simulink library "inp"  (Simulink 8.2)
settings: Solver=ode45  SolverMode=SingleTasking  StartTime=0.0  StopTime=10.0  RelTol=1e-3
undefined symbols (not defined in the file or in its folder): C_TICK_TIME_S, ENUM_FAULTSTATE_FAIL, ENUM_FAULTSTATE_INDETERMINATE, ENUM_FAULTSTATE_PASS, ENUM_KEYSWITCH_ON, FALSE, KINP_ABS1CANCommuOk_flg_ovrdflg, KINP_ABS1CANCommuOk_flg_ovrdval, KINP_ABS1CANPeriodTime_s, KINP_ABS1CANRolCntOk_flg_ovrdflg, KINP_ABS1CANRolCntOk_flg_ovrdval, KINP_ABS2CANChkSumOk_flg_ovrdflg, KINP_ABS2CANChkSumOk_flg_ovrdval, KINP_ABS2CANCommuOk_flg_ovrdflg, KINP_ABS2CANCommuOk_flg_ovrdval, KINP_ABS2CANPeriodTime_s, KINP_ABS2CANRolCntOk_flg_ovrdflg, KINP_ABS2CANRolCntOk_flg_ovrdval, KINP_ABS3CANCommuOk_flg_ovrdflg, KINP_ABS3CANCommuOk_flg_ovrdval, KINP_ABS4CANCommuOk_flg_ovrdflg, KINP_ABS4CANCommuOk_flg_ovrdval, KINP_ABSActive_flg_defval, KINP_ABSActive_flg_invalid, KINP_ABSActive_flg_ovrdflg, KINP_ABSActive_flg_ovrdval, KINP_ABSActive_flg_setvalid, KINP_ABSActive_flg_srvcflg, KINP_ABSActive_flg_srvcval, KINP_ABSCANAlive_flg_ovrdflg, KINP_ABSCANAlive_flg_ovrdval, KINP_ABSCANCommuOk_flg_ovrdflg, KINP_ABSCANCommuOk_flg_ovrdval, KINP_ABSCANValidOk_flg_ovrdflg, KINP_ABSCANValidOk_flg_ovrdval, KINP_ABSFault_flg_defval, KINP_ABSFault_flg_invalid, KINP_ABSFault_flg_ovrdflg, KINP_ABSFault_flg_ovrdval, KINP_ABSFault_flg_setvalid, KINP_ABSFault_flg_srvcflg, KINP_ABSFault_flg_srvcval, KINP_AC1CANCommuOk_flg_ovrdflg, KINP_AC1CANCommuOk_flg_ovrdval, KINP_ACCANAlive_flg_ovrdflg, KINP_ACCANAlive_flg_ovrdval, KINP_ACCANCommuOk_flg_ovrdflg, KINP_ACCANCommuOk_flg_ovrdval, KINP_ACCANValidOk_flg_ovrdflg, KINP_ACCANValidOk_flg_ovrdval, KINP_ACCCancelH_flg_defval, KINP_ACCCancelH_flg_invalid, KINP_ACCCancelH_flg_ovrdflg, KINP_ACCCancelH_flg_ovrdval, KINP_ACCCancelH_flg_setvalid, KINP_ACCCancelH_flg_srvcflg, KINP_ACCCancelH_flg_srvcval, KINP_AccelPedalPosn_pct_defval, KINP_AccelPedalPosn_pct_gain, KINP_AccelPedalPosn_pct_invalid ... (+1866)
libraries: inp x192, hcu_lib x34, simulink x33
(part 13 of 21)

== system inp/INP/HMI_signals/LFCPilotOnH ==
  signal_in : Inport
  msg_valid : Inport  Port=2
  Active_fault_flg : Inport  Port=3
  can_signal_process_wio_validity : inp/can_signal_process_wio_validity
  defval : Constant  Value=KINP_LFCPilotOnH_flg_defval
  invalid : Constant  Value=KINP_LFCPilotOnH_flg_invalid
  ovrdflg : Constant  Value=KINP_LFCPilotOnH_flg_ovrdflg
  ovrdval : Constant  Value=KINP_LFCPilotOnH_flg_ovrdval
  setvalid : Constant  Value=KINP_LFCPilotOnH_flg_setvalid
  srvcflg : Constant  Value=KINP_LFCPilotOnH_flg_srvcflg
  srvcval : Constant  Value=KINP_LFCPilotOnH_flg_srvcval
  signal_out : Outport
  signal_valid_out : Outport  Port=2
  wires:
  can_signal_process_wio_validity/1 -> signal_out/1
  can_signal_process_wio_validity/2 -> signal_valid_out/1
  signal_in/1 -> can_signal_process_wio_validity/1
  msg_valid/1 -> can_signal_process_wio_validity/2
  defval/1 -> can_signal_process_wio_validity/4
  invalid/1 -> can_signal_process_wio_validity/5
  setvalid/1 -> can_signal_process_wio_validity/6
  ovrdflg/1 -> can_signal_process_wio_validity/7
  ovrdval/1 -> can_signal_process_wio_validity/8
  srvcflg/1 -> can_signal_process_wio_validity/9
  srvcval/1 -> can_signal_process_wio_validity/10
  Active_fault_flg/1 -> can_signal_process_wio_validity/3

== system inp/INP/HMI_signals/LKAEnableH ==
  signal_in : Inport
  msg_valid : Inport  Port=2
  Active_fault_flg : Inport  Port=3
  can_signal_process_wio_validity : inp/can_signal_process_wio_validity
  defval : Constant  Value=KINP_LKAEnableH_flg_defval
  invalid : Constant  Value=KINP_LKAEnableH_flg_invalid
  ovrdflg : Constant  Value=KINP_LKAEnableH_flg_ovrdflg
  ovrdval : Constant  Value=KINP_LKAEnableH_flg_ovrdval
  setvalid : Constant  Value=KINP_LKAEnableH_flg_setvalid
  srvcflg : Constant  Value=KINP_LKAEnableH_flg_srvcflg
  srvcval : Constant  Value=KINP_LKAEnableH_flg_srvcval
  signal_out : Outport
  signal_valid_out : Outport  Port=2
  wires:
  Active_fault_flg/1 -> can_signal_process_wio_validity/3
  srvcval/1 -> can_signal_process_wio_validity/10
  srvcflg/1 -> can_signal_process_wio_validity/9
  ovrdval/1 -> can_signal_process_wio_validity/8
  ovrdflg/1 -> can_signal_process_wio_validity/7
  setvalid/1 -> can_signal_process_wio_validity/6
  invalid/1 -> can_signal_process_wio_validity/5
  defval/1 -> can_signal_process_wio_validity/4
  msg_valid/1 -> can_signal_process_wio_validity/2
  signal_in/1 -> can_signal_process_wio_validity/1
  can_signal_process_wio_validity/2 -> signal_valid_out/1
  can_signal_process_wio_validity/1 -> signal_out/1

== system inp/INP/HMI_signals/MAPAEnableH ==
  signal_in : Inport
  msg_valid : Inport  Port=2
  Active_fault_flg : Inport  Port=3
  can_signal_process_wio_validity : inp/can_signal_process_wio_validity
  defval : Constant  Value=KINP_MAPAEnableH_flg_defval
  invalid : Constant  Value=KINP_MAPAEnableH_flg_invalid
  ovrdflg : Constant  Value=KINP_MAPAEnableH_flg_ovrdflg
  ovrdval : Constant  Value=KINP_MAPAEnableH_flg_ovrdval
  setvalid : Constant  Value=KINP_MAPAEnableH_flg_setvalid
  srvcflg : Constant  Value=KINP_MAPAEnableH_flg_srvcflg
  srvcval : Constant  Value=KINP_MAPAEnableH_flg_srvcval
  signal_out : Outport
  signal_valid_out : Outport  Port=2
  wires:
  can_signal_process_wio_validity/1 -> signal_out/1
  can_signal_process_wio_validity/2 -> signal_valid_out/1
  signal_in/1 -> can_signal_process_wio_validity/1
  msg_valid/1 -> can_signal_process_wio_validity/2
  defval/1 -> can_signal_process_wio_validity/4
  invalid/1 -> can_signal_process_wio_validity/5
  setvalid/1 -> can_signal_process_wio_validity/6
  ovrdflg/1 -> can_signal_process_wio_validity/7
  ovrdval/1 -> can_signal_process_wio_validity/8
  srvcflg/1 -> can_signal_process_wio_validity/9
  srvcval/1 -> can_signal_process_wio_validity/10
  Active_fault_flg/1 -> can_signal_process_wio_validity/3

== system inp/INP/HWIO_signals/BatteryVolt ==
  signal_raw : Inport
  adc_fault : Inport  Port=2
  vref_fault : Inport  Port=3
  sample_time : Inport  Port=4
  Active_fault_flg : Inport  Port=5
  ad_signal_process : Subsystem
  defval : Constant  Value=KINP_BatteryVolt_V_defval
  limlow : Constant  Value=KINP_BatteryVolt_V_limlow
  limupr : Constant  Value=KINP_BatteryVolt_V_limupr
  ovrdflg : Constant  Value=KINP_BatteryVolt_V_ovrdflg
  ovrdval : Constant  Value=KINP_BatteryVolt_V_ovrdval
  srvcflg : Constant  Value=KINP_BatteryVolt_V_srvcflg
  srvcval : Constant  Value=KINP_BatteryVolt_V_srvcval
  tcfilt : Constant  Value=KINP_BatteryVolt_V_tcfilt
  fault_hi : Outport
  fault_lo : Outport  Port=2
  signal_out : Outport  Port=3
  signal_valid : Outport  Port=4
  wires:
  Active_fault_flg/1 -> ad_signal_process/7(Active_fault_flg)
  srvcval/1 -> ad_signal_process/13(service_value)
  srvcflg/1 -> ad_signal_process/12(service_flag)
  ovrdval/1 -> ad_signal_process/11(cal_ovrdval)
  ovrdflg/1 -> ad_signal_process/10(cal_ovrdflg)
  defval/1 -> ad_signal_process/9(def_value)
  tcfilt/1 -> ad_signal_process/8(tc)
  sample_time/1 -> ad_signal_process/6(ts)
  vref_fault/1 -> ad_signal_process/5(vref_fault)
  adc_fault/1 -> ad_signal_process/4(adc_fault)
  limlow/1 -> ad_signal_process/3(raw_lower_limit)
  limupr/1 -> ad_signal_process/2(raw_upper_limit)
  ad_signal_process/3(valid_signal) -> signal_out/1
  ad_signal_process/4(signal_valid_flg) -> signal_valid/1
  ad_signal_process/1(signal_range_high_flt) -> fault_hi/1
  ad_signal_process/2(signal_range_low_flt) -> fault_lo/1
  signal_raw/1 -> ad_signal_process/1(raw_value)

== system inp/INP/HWIO_signals/IgnitionVolt ==
  signal_raw : Inport
  VHAL_KeySwitchStatus_enum : Inport  Port=2
  adc_fault : Inport  Port=3
  vref_fault : Inport  Port=4
  sample_time : Inport  Port=5
  Active_fault_flg : Inport  Port=6
  ad_signal_process : Subsystem
  defval : Constant  Value=KINP_IgnitionVolt_V_defval
  limlow : Constant  Value=KINP_IgnitionVolt_V_limlow
  limupr : Constant  Value=KINP_IgnitionVolt_V_limupr
  ovrdflg : Constant  Value=KINP_IgnitionVolt_V_ovrdflg
  ovrdval : Constant  Value=KINP_IgnitionVolt_V_ovrdval
  srvcflg : Constant  Value=KINP_IgnitionVolt_V_srvcflg
  srvcval : Constant  Value=KINP_IgnitionVolt_V_srvcval
  tcfilt : Constant  Value=KINP_IgnitionVolt_V_tcfilt
  fault_hi : Outport
  fault_lo : Outport  Port=2
  signal_out : Outport  Port=3
  signal_valid : Outport  Port=4
  wires:
  signal_raw/1 -> ad_signal_process/1(raw_value)
  ad_signal_process/2(signal_range_low_flt) -> fault_lo/1
  ad_signal_process/1(signal_range_high_flt) -> fault_hi/1
  ad_signal_process/4(signal_valid_flg) -> signal_valid/1
  ad_signal_process/3(valid_signal) -> signal_out/1
  limupr/1 -> ad_signal_process/2(raw_upper_limit)
  limlow/1 -> ad_signal_process/3(raw_lower_limit)
  adc_fault/1 -> ad_signal_process/5(adc_fault)
  vref_fault/1 -> ad_signal_process/6(vref_fault)
  sample_time/1 -> ad_signal_process/7(ts)
  tcfilt/1 -> ad_signal_process/9(tc)
  defval/1 -> ad_signal_process/10(def_value)
  ovrdflg/1 -> ad_signal_process/11(cal_ovrdflg)
  ovrdval/1 -> ad_signal_process/12(cal_ovrdval)
  srvcflg/1 -> ad_signal_process/13(service_flag)
  srvcval/1 -> ad_signal_process/14(service_value)
  Active_fault_flg/1 -> ad_signal_process/8(Active_fault_flg)
  VHAL_KeySwitchStatus_enum/1 -> ad_signal_process/4(VHAL_KeySwitchStatus_enum)

== system inp/INP/InputSignals/Diagnosis ==
  ECM_signal_bus : Inport
  TCM_signal_bus : Inport  Port=2
  BCM_signal_bus : Inport  Port=3
  ABS_signal_bus : Inport  Port=4
  EPS_signal_bus : Inport  Port=5
  METER_signal_bus : Inport  Port=6
  AC_signal_bus : Inport  Port=7
  EPB_signal_bus : Inport  Port=8
  CSW_signal_bus : Inport  Port=9
  VCU_signal_bus : Inport  Port=10
  HMI_signal_bus : Inport  Port=11
  EPM_signal_bus : Inport  Port=12
  HWIO_signal_bus : Inport  Port=13
  LLSW_signal_bus : Inport  Port=14
  ProtCC_bus : Inport  Port=15
  CANvalid_bus : Inport  Port=16
  ABS_CANSig_Detection : Subsystem
  AC_CANSig_Detection : Subsystem
  BCM_CANSig_Detection : Subsystem
  Bus Selector1 : BusSelector  OutputSignals=VINP_CANMsgABS1CommuOk_flg,VINP_CANMsgABS2CommuOk_flg,VINP_CANMsgABS3CommuOk_flg,VINP_CANMsgABS4CommuOk_flg,VINP_CANMsgBCM1CommuOk_flg,VINP_CANMsgBCM2CommuOk_flg,VINP_CANMsgECM1CommuOk_flg,VINP_CANMsgECM2CommuOk_flg,VINP_CANMsgECM3CommuOk_flg,VINP_CANMsgECM9CommuOk_flg,VINP_CANMsgTCM1CommuOk_flg,VINP_CANMsgTCM2CommuOk_flg,VINP_CANMsgSAS1CommuOk_flg,VINP_CANMsgEPAS1CommuOk_flg,VINP_CANMsgEPAS2CommuOk_flg,VINP_CANMsgEPAS3CommuOk_flg,VINP_CANMsgMETER1CommuOk_flg,VINP_CANMsgMETER3CommuOk_flg,VINP_CANMsgAC1CommuOk_flg,VINP_CANMsgEPB1CommuOk_flg,VINP_CANMsgVCU1CommuOk_flg,VINP_CANMsgVCU2CommuOk_flg,VINP_CANMsgHCU3CommuOk_flg,VINP_CANMsgHCU4CommuOk_flg,VINP_CANMsgHMI2CommuOk_flg,VINP_CANMsgHMI1CommuOk_flg,VINP_CANMsgCSW1CommuOk_flg,VINP_CANMsgEPMCommuOk_flg
  Bus Selector10 : BusSelector  OutputSignals=VINP_OutsideTempSigOk_flg_InHAL
  Bus Selector11 : BusSelector  OutputSignals=VINP_BrkLampOnSigOk_flg_InHAL,VINP_VehSpdSigOk_flg_InHAL,VINP_YawRateSigOk_flg_InHAL,VINP_LatAccelSigOk_flg_InHAL,VINP_LongAccelSigOk_flg_InHAL,VINP_MCPressureSigOk_flg_InHAL,VINP_SurfaceGradeSigOk_flg_InHAL,VINP_BrakBoosterPresSigOk_flg_InHAL,VINP_LFWheelSpeedSigOk_flg_InHAL,VINP_LRWheelSpeedSigOk_flg_InHAL,VINP_RFWheelSpeedSigOk_flg_InHAL,VINP_RRWheelSpeedSigOk_flg_InHAL
  Bus Selector12 : BusSelector  OutputSignals=VINP_IgnitionVoltFltRangeHigh_enum_InHAL,VINP_IgnitionVoltFltRangeLow_enum_InHAL,VINP_BatteryVoltFltRangeHigh_enum_InHAL,VINP_BatteryVoltFltRangeLow_enum_InHAL
  Bus Selector13 : BusSelector  OutputSignals=VHAL_ADCFault_enum,VHAL_EEPROMFault_enum,VHAL_RAMFault_enum,VHAL_ROMFault_enum,VHAL_VREF1Fault_enum,VHAL_VREF2Fault_enum
  Bus Selector14 : BusSelector  OutputSignals=VINP_SteerAngleSigOk_flg_InHAL,VINP_SteerSpeedSigOk_flg_InHAL,VINP_RelSteerAngleSigOk_flg_InHAL,VINP_SteerTorqueSigOk_flg_InHAL,VINP_AckCurStrAngReqSigOk_flg_InHAL,VINP_AckCurStrTqReqSigOk_flg_InHAL,VINP_EPSBattVoltSigOk_flg_InHAL
  Bus Selector15 : BusSelector  OutputSignals=VINP_OdoMeterSigOk_flg_InHAL,VINP_MeterVehSpdSigOk_flg_InHAL
  Bus Selector16 : BusSelector  OutputSignals=VINP_ParkBrakeBindStaSigOk_flg_InHAL
  Bus Selector17 : BusSelector  OutputSignals=VINP_AckCurBrakDecReqSigOk_flg_InHAL,VINP_AckCurPTTqReqSigOk_flg_InHAL,VINP_AckCurVehDecSigOk_flg_InHAL,VINP_DriverBrakPedalOnSigOk_flg_InHAL,VINP_ESCBattVoltSigOk_flg_InHAL,VINP_HWAccelPedalPosnSigOk_flg_InHAL,VINP_CurDriverTqReqSigOk_flg_InHAL
  Bus Selector2 : BusSelector  OutputSignals=VINP_VehCrashedSigOk_flg_InHAL
  Bus Selector26 : BusSelector  OutputSignals=VINP_CANMsgABS1CommuOk_flg,VINP_CANMsgABS2CommuOk_flg,VINP_CANMsgABS3CommuOk_flg,VINP_CANMsgABS4CommuOk_flg,VINP_CANMsgBCM1CommuOk_flg,VINP_CANMsgBCM2CommuOk_flg,VINP_CANMsgECM1CommuOk_flg,VINP_CANMsgECM2CommuOk_flg,VINP_CANMsgECM3CommuOk_flg,VINP_CANMsgECM9CommuOk_flg,VINP_CANMsgTCM1CommuOk_flg,VINP_CANMsgTCM2CommuOk_flg,VINP_CANMsgSAS1CommuOk_flg,VINP_CANMsgEPAS1CommuOk_flg,VINP_CANMsgEPAS2CommuOk_flg,VINP_CANMsgEPAS3CommuOk_flg,VINP_CANMsgMETER1CommuOk_flg,VINP_CANMsgMETER3CommuOk_flg,VINP_CANMsgAC1CommuOk_flg,VINP_CANMsgEPB1CommuOk_flg,VINP_CANMsgVCU1CommuOk_flg,VINP_CANMsgVCU2CommuOk_flg,VINP_CANMsgHCU3CommuOk_flg,VINP_CANMsgHCU4CommuOk_flg,VINP_CANMsgHMI2CommuOk_flg,VINP_CANMsgHMI1CommuOk_flg,VINP_CANMsgCSW1CommuOk_flg,VINP_CANMsgEPMCommuOk_flg
  Bus Selector3 : BusSelector  OutputSignals=VINP_ShifterPosnSigOk_flg_InHAL,VINP_NowGearPosnSigOk_flg_InHAL,VINP_TMTurbineSpdSigOk_flg_InHAL
  Bus Selector4 : BusSelector  OutputSignals=VINP_CANMsgABS1RolCntOk_flg,VINP_CANMsgABS2RolCntOk_flg,VINP_CANMsgECM9RolCntOk_flg,VINP_CANMsgSAS1RolCntOk_flg,VINP_CANMsgEPAS2RolCntOk_flg,VINP_CANMsgEPAS3RolCntOk_flg,VINP_CANMsgVCU1RolCntOk_flg,VINP_CANMsgVCU2RolCntOk_flg,VINP_CANMsgHCU3RolCntOk_flg,VINP_CANMsgABS2ChkSumOk_flg,VINP_CANMsgSAS1ChkSumOk_flg,VINP_CANMsgEPAS2ChkSumOk_flg,VINP_CANMsgEPAS3ChkSumOk_flg,VINP_CANMsgVCU1ChkSumOk_flg,VINP_CANMsgVCU2ChkSumOk_flg,VINP_CamCANReceiveOk_flg
  Bus Selector5 : BusSelector  OutputSignals=VINP_CANMsgABS1CommuOk_flg,VINP_CANMsgABS2CommuOk_flg,VINP_CANMsgABS3CommuOk_flg,VINP_CANMsgABS4CommuOk_flg,VINP_CANMsgBCM1CommuOk_flg,VINP_CANMsgBCM2CommuOk_flg,VINP_CANMsgECM1CommuOk_flg,VINP_CANMsgECM2CommuOk_flg,VINP_CANMsgECM3CommuOk_flg,VINP_CANMsgECM9CommuOk_flg,VINP_CANMsgTCM1CommuOk_flg,VINP_CANMsgTCM2CommuOk_flg,VINP_CANMsgSAS1CommuOk_flg,VINP_CANMsgEPAS1CommuOk_flg,VINP_CANMsgEPAS2CommuOk_flg,VINP_CANMsgEPAS3CommuOk_flg,VINP_CANMsgMETER1CommuOk_flg,VINP_CANMsgMETER3CommuOk_flg,VINP_CANMsgAC1CommuOk_flg,VINP_CANMsgEPB1CommuOk_flg,VINP_CANMsgVCU1CommuOk_flg,VINP_CANMsgVCU2CommuOk_flg,VINP_CANMsgHCU3CommuOk_flg,VINP_CANMsgHCU4CommuOk_flg,VINP_CANMsgHMI2CommuOk_flg,VINP_CANMsgHMI1CommuOk_flg,VINP_CANMsgCSW1CommuOk_flg,VINP_CANMsgEPMCommuOk_flg
  Bus Selector6 : BusSelector  OutputSignals=VINP_CANMsgABS1CommuOk_flg,VINP_CANMsgABS2CommuOk_flg,VINP_CANMsgABS3CommuOk_flg,VINP_CANMsgABS4CommuOk_flg,VINP_CANMsgBCM1CommuOk_flg,VINP_CANMsgBCM2CommuOk_flg,VINP_CANMsgECM1CommuOk_flg,VINP_CANMsgECM2CommuOk_flg,VINP_CANMsgECM3CommuOk_flg,VINP_CANMsgECM9CommuOk_flg,VINP_CANMsgTCM1CommuOk_flg,VINP_CANMsgTCM2CommuOk_flg,VINP_CANMsgSAS1CommuOk_flg,VINP_CANMsgEPAS1CommuOk_flg,VINP_CANMsgEPAS2CommuOk_flg,VINP_CANMsgEPAS3CommuOk_flg,VINP_CANMsgMETER1CommuOk_flg,VINP_CANMsgMETER3CommuOk_flg,VINP_CANMsgAC1CommuOk_flg,VINP_CANMsgEPB1CommuOk_flg,VINP_CANMsgVCU1CommuOk_flg,VINP_CANMsgVCU2CommuOk_flg,VINP_CANMsgHCU3CommuOk_flg,VINP_CANMsgHCU4CommuOk_flg,VINP_CANMsgHMI2CommuOk_flg,VINP_CANMsgHMI1CommuOk_flg,VINP_CANMsgCSW1CommuOk_flg,VINP_CANMsgEPMCommuOk_flg,VINP_CamCANReceiveOk_flg,VINP_CANMsgECM9RolCntOk_flg,VINP_CANMsgABS1RolCntOk_flg,VINP_CANMsgABS2RolCntOk_flg,VINP_CANMsgSAS1RolCntOk_flg,VINP_CANMsgEPAS2RolCntOk_flg,VINP_CANMsgEPAS3RolCntOk_flg,VINP_CANMsgVCU1RolCntOk_flg,VINP_CANMsgVCU2RolCntOk_flg,VINP_CANMsgHCU3RolCntOk_flg,VINP_CANMsgABS2ChkSumOk_flg,VINP_CANMsgSAS1ChkSumOk_flg,VINP_CANMsgEPAS2ChkSumOk_flg,VINP_CANMsgEPAS3ChkSumOk_flg,VINP_CANMsgVCU1ChkSumOk_flg,VINP_CANMsgVCU2ChkSumOk_flg
  Bus Selector9 : BusSelector  OutputSignals=VINP_AccelPedalPosnSigOk_flg_InHAL,VINP_EffAccelPedalPosnSigOk_flg_InHAL,VINP_EngSpdSigOk_flg_InHAL,VINP_EngTqFrictionSigOk_flg_InHAL,VINP_EngTqIndicatedSigOk_flg_InHAL,VINP_EngTqIndicatedCORSigOk_flg_InHAL,VINP_EngTqMaxSigOk_flg_InHAL,VINP_EngTqMinSigOk_flg_InHAL
  CANCommunicationDetection : Subsystem
  CANCommunicationDetection_byID : Subsystem
  CANInvalidDetection : Subsystem
  CANInvalidDetection_byType : Subsystem
  ECM_CANSig_Detection : Subsystem
  EPB_CANSig_Detection : Subsystem
  EPS_CANSig_Detection : Subsystem
  HWIO_Detection : Subsystem
  LLSW_Detection : Subsystem
  METER_CANSig_Detection : Subsystem
  Proc_CANAlive : Subsystem
  TCM_CANSig_Detection : Subsystem
  Terminator{,1..15} (16 alike) : Terminator
  VCU_CANSig_Detection : Subsystem
  inputs_bus2 : BusCreator  Inputs=24
  INP_diag_bus : Outport
  wires:
  [LLSW_signal_bus] -> Terminator8/1
  [HWIO_signal_bus] -> Terminator7/1
  [EPM_signal_bus] -> Terminator6/1
  [EPS_signal_bus] -> Terminator4/1
  [ABS_signal_bus] -> Terminator3/1
  [BCM_signal_bus] -> Terminator2/1
  [TCM_signal_bus] -> Terminator1/1
  [ECM_signal_bus] -> Terminator/1
  ECM_signal_bus/1 -> [ECM_signal_bus]
  TCM_signal_bus/1 -> [TCM_signal_bus]
  BCM_signal_bus/1 -> [BCM_signal_bus]
  ABS_signal_bus/1 -> [ABS_signal_bus]
  EPS_signal_bus/1 -> [EPS_signal_bus]
  EPM_signal_bus/1 -> [EPM_signal_bus]
  HWIO_signal_bus/1 -> [HWIO_signal_bus]
  LLSW_signal_bus/1 -> [LLSW_signal_bus]
  ProtCC_bus/1 -> [ProtCC_bus]
  CANvalid_bus/1 -> [CANvalid_bus]
  [CANvalid_bus] -> Terminator10/1
  [ProtCC_bus] -> Terminator9/1
  [HWIO_signal_bus] -> Bus Selector12/1
  Bus Selector12/1 -> HWIO_Detection/1(VINP_IgnitionVoltFltRangeHigh_enum_InHAL)  "<VINP_IgnitionVoltFltRangeHigh_enum_InHAL>"
  Bus Selector12/2 -> HWIO_Detection/2(VINP_IgnitionVoltFltRangeLow_enum_InHAL)  "<VINP_IgnitionVoltFltRangeLow_enum_InHAL>"
  [LLSW_signal_bus] -> Bus Selector13/1
  Bus Selector13/2 -> LLSW_Detection/2(VHAL_EEPROMFault_enum)  "<VHAL_EEPROMFault_enum>"
  Bus Selector13/3 -> LLSW_Detection/3(VHAL_RAMFault_enum)  "<VHAL_RAMFault_enum>"
  Bus Selector13/4 -> LLSW_Detection/4(VHAL_ROMFault_enum)  "<VHAL_ROMFault_enum>"
  Bus Selector13/5 -> LLSW_Detection/5(VHAL_VREF1Fault_enum)  "<VHAL_VREF1Fault_enum>"
  Bus Selector13/6 -> LLSW_Detection/6(VHAL_VREF2Fault_enum)  "<VHAL_VREF2Fault_enum>"
  Bus Selector13/1 -> LLSW_Detection/1(VHAL_ADCFault_enum)  "<VHAL_ADCFault_enum>"
  Bus Selector12/3 -> HWIO_Detection/3(VINP_BatteryVoltFltRangeHigh_enum_InHAL)  "<VINP_BatteryVoltFltRangeHigh_enum_InHAL>"
  Bus Selector12/4 -> HWIO_Detection/4(VINP_BatteryVoltFltRangeLow_enum_InHAL)  "<VINP_BatteryVoltFltRangeLow_enum_InHAL>"
  [METER_signal_bus] -> Terminator14/1
  METER_signal_bus/1 -> [METER_signal_bus]
  [AC_signal_bus] -> Terminator15/1
  AC_signal_bus/1 -> [AC_signal_bus]
  [EPB_signal_bus] -> Terminator11/1
  EPB_signal_bus/1 -> [EPB_signal_bus]
  [VCU_signal_bus] -> Terminator12/1
  VCU_signal_bus/1 -> [VCU_signal_bus]
  [HMI_signal_bus] -> Terminator13/1
  HMI_signal_bus/1 -> [HMI_signal_bus]
  CANCommunicationDetection/5(VINP_EPSCANValid_flg_InProc) -> [VINP_EPSCANValid_flg_InProc]
  CANCommunicationDetection/4(VINP_TCMCANValid_flg_InProc) -> [VINP_TCMCANValid_flg_InProc]
  CANCommunicationDetection/3(VINP_ECMCANValid_flg_InProc) -> [VINP_ECMCANValid_flg_InProc]
  CANCommunicationDetection/2(VINP_BCMCANValid_flg_InProc) -> [VINP_BCMCANValid_flg_InProc]
  CANCommunicationDetection/1(VINP_ABSCANValid_flg_InProc) -> [VINP_ABSCANValid_flg_InProc]
  Bus Selector26/13 -> CANCommunicationDetection/13(VINP_CANMsgSAS1CommuOk_flg)  "<VINP_CANMsgSAS1CommuOk_flg>"
  Bus Selector26/11 -> CANCommunicationDetection/11(VINP_CANMsgTCM1CommuOk_flg)  "<VINP_CANMsgTCM1CommuOk_flg>"
  Bus Selector26/10 -> CANCommunicationDetection/10(VINP_CANMsgECM9CommuOk_flg)  "<VINP_CANMsgECM9CommuOk_flg>"
  Bus Selector26/9 -> CANCommunicationDetection/9(VINP_CANMsgECM3CommuOk_flg)  "<VINP_CANMsgECM3CommuOk_flg>"
  Bus Selector26/5 -> CANCommunicationDetection/5(VINP_CANMsgBCM1CommuOk_flg)  "<VINP_CANMsgBCM1CommuOk_flg>"
  Bus Selector26/4 -> CANCommunicationDetection/4(VINP_CANMsgABS4CommuOk_flg)  "<VINP_CANMsgABS4CommuOk_flg>"
  Bus Selector26/2 -> CANCommunicationDetection/2(VINP_CANMsgABS2CommuOk_flg)  "<VINP_CANMsgABS2CommuOk_flg>"
  [CANvalid_bus] -> Bus Selector26/1
  Bus Selector26/15 -> CANCommunicationDetection/15(VINP_CANMsgEPAS2CommuOk_flg)  "<VINP_CANMsgEPAS2CommuOk_flg>"
  Bus Selector26/16 -> CANCommunicationDetection/16(VINP_CANMsgEPAS3CommuOk_flg)  "<VINP_CANMsgEPAS3CommuOk_flg>"
  Bus Selector26/17 -> CANCommunicationDetection/17(VINP_CANMsgMETER1CommuOk_flg)  "<VINP_CANMsgMETER1CommuOk_flg>"
  CANCommunicationDetection/6(VINP_METERCANValid_flg_InProc) -> [VINP_METERCANValid_flg_InProc]
  Bus Selector26/7 -> CANCommunicationDetection/7(VINP_CANMsgECM1CommuOk_flg)  "<VINP_CANMsgECM1CommuOk_flg>"
  Bus Selector26/1 -> CANCommunicationDetection/1(VINP_CANMsgABS1CommuOk_flg)  "<VINP_CANMsgABS1CommuOk_flg>"
  Bus Selector26/3 -> CANCommunicationDetection/3(VINP_CANMsgABS3CommuOk_flg)  "<VINP_CANMsgABS3CommuOk_flg>"
  Bus Selector26/6 -> CANCommunicationDetection/6(VINP_CANMsgBCM2CommuOk_flg)  "<VINP_CANMsgBCM2CommuOk_flg>"
  Bus Selector26/8 -> CANCommunicationDetection/8(VINP_CANMsgECM2CommuOk_flg)  "<VINP_CANMsgECM2CommuOk_flg>"
  Bus Selector26/12 -> CANCommunicationDetection/12(VINP_CANMsgTCM2CommuOk_flg)  "<VINP_CANMsgTCM2CommuOk_flg>"
  Bus Selector26/18 -> CANCommunicationDetection/18(VINP_CANMsgMETER3CommuOk_flg)  "<VINP_CANMsgMETER3CommuOk_flg>"
  Bus Selector26/19 -> CANCommunicationDetection/19(VINP_CANMsgAC1CommuOk_flg)  "<VINP_CANMsgAC1CommuOk_flg>"
  Bus Selector26/20 -> CANCommunicationDetection/20(VINP_CANMsgEPB1CommuOk_flg)  "<VINP_CANMsgEPB1CommuOk_flg>"
  Bus Selector26/21 -> CANCommunicationDetection/21(VINP_CANMsgVCU1CommuOk_flg)  "<VINP_CANMsgVCU1CommuOk_flg>"
  Bus Selector26/22 -> CANCommunicationDetection/22(VINP_CANMsgVCU2CommuOk_flg)  "<VINP_CANMsgVCU2CommuOk_flg>"
  Bus Selector26/23 -> CANCommunicationDetection/23(VINP_CANMsgHCU3CommuOk_flg)  "<VINP_CANMsgHCU3CommuOk_flg>"
  CANCommunicationDetection/7(VINP_ACCANValid_flg_InProc) -> [VINP_ACCANValid_flg_InProc]
  CANCommunicationDetection/8(VINP_EPBCANValid_flg_InProc) -> [VINP_EPBCANValid_flg_InProc]
  CANCommunicationDetection/9(VINP_VCUCANValid_flg_InProc) -> [VINP_VCUCANValid_flg_InProc]
  Bus Selector26/24 -> CANCommunicationDetection/24(VINP_CANMsgHCU4CommuOk_flg)  "<VINP_CANMsgHCU4CommuOk_flg>"
  Bus Selector26/25 -> CANCommunicationDetection/25(VINP_CANMsgHMI2CommuOk_flg)  "<VINP_CANMsgHMI2CommuOk_flg>"
  Bus Selector26/26 -> CANCommunicationDetection/26(VINP_CANMsgHMI1CommuOk_flg)  "<VINP_CANMsgHMI1CommuOk_flg>"
  Bus Selector26/28 -> CANCommunicationDetection/28(VINP_CANMsgEPMCommuOk_flg)  "<VINP_CANMsgEPMCommuOk_flg>"
  CANCommunicationDetection/10(VINP_HMICANValid_flg_InProc) -> [VINP_HMICANValid_flg_InProc]
  CANCommunicationDetection/12(VINP_EPMCANValid_flg_InProc) -> [VINP_EPMCANValid_flg_InProc]
  Proc_CANAlive/5(VINP_EPSCANAlive_flg_InProc) -> [VINP_EPSCANAlive_flg_InProc]
  Proc_CANAlive/4(VINP_TCMCANAlive_flg_InProc) -> [VINP_TCMCANAlive_flg_InProc]
  Proc_CANAlive/3(VINP_ECMCANAlive_flg_InProc) -> [VINP_ECMCANAlive_flg_InProc]
  Proc_CANAlive/2(VINP_BCMCANAlive_flg_InProc) -> [VINP_BCMCANAlive_flg_InProc]
  Proc_CANAlive/1(VINP_ABSCANAlive_flg_InProc) -> [VINP_ABSCANAlive_flg_InProc]
  Bus Selector1/13 -> Proc_CANAlive/13(VINP_CANMsgSAS1CommuOk_flg)  "<VINP_CANMsgSAS1CommuOk_flg>"
  Bus Selector1/11 -> Proc_CANAlive/11(VINP_CANMsgTCM1CommuOk_flg)  "<VINP_CANMsgTCM1CommuOk_flg>"
  Bus Selector1/10 -> Proc_CANAlive/10(VINP_CANMsgECM9CommuOk_flg)  "<VINP_CANMsgECM9CommuOk_flg>"
  Bus Selector1/9 -> Proc_CANAlive/9(VINP_CANMsgECM3CommuOk_flg)  "<VINP_CANMsgECM3CommuOk_flg>"
  Bus Selector1/5 -> Proc_CANAlive/5(VINP_CANMsgBCM1CommuOk_flg)  "<VINP_CANMsgBCM1CommuOk_flg>"
  Bus Selector1/4 -> Proc_CANAlive/4(VINP_CANMsgABS4CommuOk_flg)  "<VINP_CANMsgABS4CommuOk_flg>"
  Bus Selector1/2 -> Proc_CANAlive/2(VINP_CANMsgABS2CommuOk_flg)  "<VINP_CANMsgABS2CommuOk_flg>"
  [CANvalid_bus] -> Bus Selector1/1
  Bus Selector1/15 -> Proc_CANAlive/15(VINP_CANMsgEPAS2CommuOk_flg)  "<VINP_CANMsgEPAS2CommuOk_flg>"
  Bus Selector1/16 -> Proc_CANAlive/16(VINP_CANMsgEPAS3CommuOk_flg)  "<VINP_CANMsgEPAS3CommuOk_flg>"
  Bus Selector1/17 -> Proc_CANAlive/17(VINP_CANMsgMETER1CommuOk_flg)  "<VINP_CANMsgMETER1CommuOk_flg>"
  Proc_CANAlive/6(VINP_METERCANAlive_flg_InProc) -> [VINP_METERCANAlive_flg_InProc]
  Bus Selector1/7 -> Proc_CANAlive/7(VINP_CANMsgECM1CommuOk_flg)  "<VINP_CANMsgECM1CommuOk_flg>"
  Bus Selector1/1 -> Proc_CANAlive/1(VINP_CANMsgABS1CommuOk_flg)  "<VINP_CANMsgABS1CommuOk_flg>"
  Bus Selector1/3 -> Proc_CANAlive/3(VINP_CANMsgABS3CommuOk_flg)  "<VINP_CANMsgABS3CommuOk_flg>"
  Bus Selector1/6 -> Proc_CANAlive/6(VINP_CANMsgBCM2CommuOk_flg)  "<VINP_CANMsgBCM2CommuOk_flg>"
  Bus Selector1/8 -> Proc_CANAlive/8(VINP_CANMsgECM2CommuOk_flg)  "<VINP_CANMsgECM2CommuOk_flg>"
  Bus Selector1/12 -> Proc_CANAlive/12(VINP_CANMsgTCM2CommuOk_flg)  "<VINP_CANMsgTCM2CommuOk_flg>"
  Bus Selector1/18 -> Proc_CANAlive/18(VINP_CANMsgMETER3CommuOk_flg)  "<VINP_CANMsgMETER3CommuOk_flg>"
  Bus Selector1/19 -> Proc_CANAlive/19(VINP_CANMsgAC1CommuOk_flg)  "<VINP_CANMsgAC1CommuOk_flg>"
  Bus Selector1/20 -> Proc_CANAlive/20(VINP_CANMsgEPB1CommuOk_flg)  "<VINP_CANMsgEPB1CommuOk_flg>"
  Bus Selector1/21 -> Proc_CANAlive/21(VINP_CANMsgVCU1CommuOk_flg)  "<VINP_CANMsgVCU1CommuOk_flg>"
  Bus Selector1/22 -> Proc_CANAlive/22(VINP_CANMsgVCU2CommuOk_flg)  "<VINP_CANMsgVCU2CommuOk_flg>"
  Bus Selector1/23 -> Proc_CANAlive/23(VINP_CANMsgHCU3CommuOk_flg)  "<VINP_CANMsgHCU3CommuOk_flg>"
  Proc_CANAlive/7(VINP_ACCANAlive_flg_InProc) -> [VINP_ACCANAlive_flg_InProc]
  Proc_CANAlive/8(VINP_EPBCANAlive_flg_InProc) -> [VINP_EPBCANAlive_flg_InProc]
  Proc_CANAlive/9(VINP_VCUCANAlive_flg_InProc) -> [VINP_VCUCANAlive_flg_InProc]
  Bus Selector1/24 -> Proc_CANAlive/24(VINP_CANMsgHCU4CommuOk_flg)  "<VINP_CANMsgHCU4CommuOk_flg>"
  Bus Selector1/25 -> Proc_CANAlive/25(VINP_CANMsgHMI2CommuOk_flg)  "<VINP_CANMsgHMI2CommuOk_flg>"
  Bus Selector1/26 -> Proc_CANAlive/26(VINP_CANMsgHMI1CommuOk_flg)  "<VINP_CANMsgHMI1CommuOk_flg>"
  Bus Selector1/28 -> Proc_CANAlive/28(VINP_CANMsgEPMCommuOk_flg)  "<VINP_CANMsgEPMCommuOk_flg>"
  Proc_CANAlive/10(VINP_HMICANAlive_flg_InProc) -> [VINP_HMICANAlive_flg_InProc]
  Proc_CANAlive/12(VINP_EPMCANAlive_flg_InProc) -> [VINP_EPMCANAlive_flg_InProc]
  Bus Selector5/10 -> CANCommunicationDetection_byID/10(VINP_CANMsgECM9CommuOk_flg)  "<VINP_CANMsgECM9CommuOk_flg>"
  Bus Selector5/9 -> CANCommunicationDetection_byID/9(VINP_CANMsgECM3CommuOk_flg)  "<VINP_CANMsgECM3CommuOk_flg>"
  Bus Selector5/4 -> CANCommunicationDetection_byID/4(VINP_CANMsgABS4CommuOk_flg)  "<VINP_CANMsgABS4CommuOk_flg>"
  Bus Selector5/2 -> CANCommunicationDetection_byID/2(VINP_CANMsgABS2CommuOk_flg)  "<VINP_CANMsgABS2CommuOk_flg>"
  [CANvalid_bus] -> Bus Selector5/1
  Bus Selector5/13 -> CANCommunicationDetection_byID/13(VINP_CANMsgSAS1CommuOk_flg)  "<VINP_CANMsgSAS1CommuOk_flg>"
  Bus Selector5/15 -> CANCommunicationDetection_byID/15(VINP_CANMsgEPAS2CommuOk_flg)  "<VINP_CANMsgEPAS2CommuOk_flg>"
  Bus Selector5/16 -> CANCommunicationDetection_byID/16(VINP_CANMsgEPAS3CommuOk_flg)  "<VINP_CANMsgEPAS3CommuOk_flg>"
  Bus Selector5/17 -> CANCommunicationDetection_byID/17(VINP_CANMsgMETER1CommuOk_flg)  "<VINP_CANMsgMETER1CommuOk_flg>"
  Bus Selector5/7 -> CANCommunicationDetection_byID/7(VINP_CANMsgECM1CommuOk_flg)  "<VINP_CANMsgECM1CommuOk_flg>"
  Bus Selector5/1 -> CANCommunicationDetection_byID/1(VINP_CANMsgABS1CommuOk_flg)  "<VINP_CANMsgABS1CommuOk_flg>"
  Bus Selector5/3 -> CANCommunicationDetection_byID/3(VINP_CANMsgABS3CommuOk_flg)  "<VINP_CANMsgABS3CommuOk_flg>"
  Bus Selector5/5 -> CANCommunicationDetection_byID/5(VINP_CANMsgBCM1CommuOk_flg)  "<VINP_CANMsgBCM1CommuOk_flg>"
  Bus Selector5/6 -> CANCommunicationDetection_byID/6(VINP_CANMsgBCM2CommuOk_flg)  "<VINP_CANMsgBCM2CommuOk_flg>"
  Bus Selector5/8 -> CANCommunicationDetection_byID/8(VINP_CANMsgECM2CommuOk_flg)  "<VINP_CANMsgECM2CommuOk_flg>"
  Bus Selector5/11 -> CANCommunicationDetection_byID/11(VINP_CANMsgTCM1CommuOk_flg)  "<VINP_CANMsgTCM1CommuOk_flg>"
  Bus Selector5/12 -> CANCommunicationDetection_byID/12(VINP_CANMsgTCM2CommuOk_flg)  "<VINP_CANMsgTCM2CommuOk_flg>"
  Bus Selector5/18 -> CANCommunicationDetection_byID/18(VINP_CANMsgMETER3CommuOk_flg)  "<VINP_CANMsgMETER3CommuOk_flg>"
  Bus Selector5/19 -> CANCommunicationDetection_byID/19(VINP_CANMsgAC1CommuOk_flg)  "<VINP_CANMsgAC1CommuOk_flg>"
  Bus Selector5/20 -> CANCommunicationDetection_byID/20(VINP_CANMsgEPB1CommuOk_flg)  "<VINP_CANMsgEPB1CommuOk_flg>"
  Bus Selector5/21 -> CANCommunicationDetection_byID/21(VINP_CANMsgVCU1CommuOk_flg)  "<VINP_CANMsgVCU1CommuOk_flg>"
  Bus Selector5/22 -> CANCommunicationDetection_byID/22(VINP_CANMsgVCU2CommuOk_flg)  "<VINP_CANMsgVCU2CommuOk_flg>"
  Bus Selector5/23 -> CANCommunicationDetection_byID/23(VINP_CANMsgHCU3CommuOk_flg)  "<VINP_CANMsgHCU3CommuOk_flg>"
  Bus Selector5/24 -> CANCommunicationDetection_byID/24(VINP_CANMsgHCU4CommuOk_flg)  "<VINP_CANMsgHCU4CommuOk_flg>"
  Bus Selector5/25 -> CANCommunicationDetection_byID/25(VINP_CANMsgHMI2CommuOk_flg)  "<VINP_CANMsgHMI2CommuOk_flg>"
  Bus Selector5/26 -> CANCommunicationDetection_byID/26(VINP_CANMsgHMI1CommuOk_flg)  "<VINP_CANMsgHMI1CommuOk_flg>"
  Bus Selector5/28 -> CANCommunicationDetection_byID/28(VINP_CANMsgEPMCommuOk_flg)  "<VINP_CANMsgEPMCommuOk_flg>"
  Bus Selector6/13 -> CANInvalidDetection/13(VINP_CANMsgSAS1CommuOk_flg)  "<VINP_CANMsgSAS1CommuOk_flg>"
  Bus Selector6/11 -> CANInvalidDetection/11(VINP_CANMsgTCM1CommuOk_flg)  "<VINP_CANMsgTCM1CommuOk_flg>"
  Bus Selector6/10 -> CANInvalidDetection/10(VINP_CANMsgECM9CommuOk_flg)  "<VINP_CANMsgECM9CommuOk_flg>"
  Bus Selector6/9 -> CANInvalidDetection/9(VINP_CANMsgECM3CommuOk_flg)  "<VINP_CANMsgECM3CommuOk_flg>"
  Bus Selector6/5 -> CANInvalidDetection/5(VINP_CANMsgBCM1CommuOk_flg)  "<VINP_CANMsgBCM1CommuOk_flg>"
  Bus Selector6/4 -> CANInvalidDetection/4(VINP_CANMsgABS4CommuOk_flg)  "<VINP_CANMsgABS4CommuOk_flg>"
  Bus Selector6/2 -> CANInvalidDetection/2(VINP_CANMsgABS2CommuOk_flg)  "<VINP_CANMsgABS2CommuOk_flg>"
  [CANvalid_bus] -> Bus Selector6/1
  Bus Selector6/15 -> CANInvalidDetection/15(VINP_CANMsgEPAS2CommuOk_flg)  "<VINP_CANMsgEPAS2CommuOk_flg>"
  Bus Selector6/16 -> CANInvalidDetection/16(VINP_CANMsgEPAS3CommuOk_flg)  "<VINP_CANMsgEPAS3CommuOk_flg>"
  Bus Selector6/17 -> CANInvalidDetection/17(VINP_CANMsgMETER1CommuOk_flg)  "<VINP_CANMsgMETER1CommuOk_flg>"
  Bus Selector6/7 -> CANInvalidDetection/7(VINP_CANMsgECM1CommuOk_flg)  "<VINP_CANMsgECM1CommuOk_flg>"
  Bus Selector6/1 -> CANInvalidDetection/1(VINP_CANMsgABS1CommuOk_flg)  "<VINP_CANMsgABS1CommuOk_flg>"
  Bus Selector6/3 -> CANInvalidDetection/3(VINP_CANMsgABS3CommuOk_flg)  "<VINP_CANMsgABS3CommuOk_flg>"
  Bus Selector6/6 -> CANInvalidDetection/6(VINP_CANMsgBCM2CommuOk_flg)  "<VINP_CANMsgBCM2CommuOk_flg>"
  Bus Selector6/8 -> CANInvalidDetection/8(VINP_CANMsgECM2CommuOk_flg)  "<VINP_CANMsgECM2CommuOk_flg>"
  Bus Selector6/12 -> CANInvalidDetection/12(VINP_CANMsgTCM2CommuOk_flg)  "<VINP_CANMsgTCM2CommuOk_flg>"
  Bus Selector6/18 -> CANInvalidDetection/18(VINP_CANMsgMETER3CommuOk_flg)  "<VINP_CANMsgMETER3CommuOk_flg>"
  Bus Selector6/19 -> CANInvalidDetection/19(VINP_CANMsgAC1CommuOk_flg)  "<VINP_CANMsgAC1CommuOk_flg>"
  Bus Selector6/20 -> CANInvalidDetection/20(VINP_CANMsgEPB1CommuOk_flg)  "<VINP_CANMsgEPB1CommuOk_flg>"
  Bus Selector6/21 -> CANInvalidDetection/21(VINP_CANMsgVCU1CommuOk_flg)  "<VINP_CANMsgVCU1CommuOk_flg>"
  Bus Selector6/22 -> CANInvalidDetection/22(VINP_CANMsgVCU2CommuOk_flg)  "<VINP_CANMsgVCU2CommuOk_flg>"
  Bus Selector6/23 -> CANInvalidDetection/23(VINP_CANMsgHCU3CommuOk_flg)  "<VINP_CANMsgHCU3CommuOk_flg>"
  Bus Selector6/24 -> CANInvalidDetection/24(VINP_CANMsgHCU4CommuOk_flg)  "<VINP_CANMsgHCU4CommuOk_flg>"
  Bus Selector6/25 -> CANInvalidDetection/25(VINP_CANMsgHMI2CommuOk_flg)  "<VINP_CANMsgHMI2CommuOk_flg>"
  Bus Selector6/26 -> CANInvalidDetection/26(VINP_CANMsgHMI1CommuOk_flg)  "<VINP_CANMsgHMI1CommuOk_flg>"
  Bus Selector6/28 -> CANInvalidDetection/28(VINP_CANMsgEPMCommuOk_flg)  "<VINP_CANMsgEPMCommuOk_flg>"
  Bus Selector6/29 -> CANInvalidDetection/29(VINP_CamCANReceiveOk_flg)  "<VINP_CamCANReceiveOk_flg>"
  Bus Selector6/30 -> CANInvalidDetection/30(VINP_CANMsgECM9RolCntOk_flg)  "<VINP_CANMsgECM9RolCntOk_flg>"
  Bus Selector6/31 -> CANInvalidDetection/31(VINP_CANMsgABS1RolCntOk_flg)  "<VINP_CANMsgABS1RolCntOk_flg>"
  Bus Selector6/32 -> CANInvalidDetection/32(VINP_CANMsgABS2RolCntOk_flg)  "<VINP_CANMsgABS2RolCntOk_flg>"
  Bus Selector6/33 -> CANInvalidDetection/33(VINP_CANMsgSAS1RolCntOk_flg)  "<VINP_CANMsgSAS1RolCntOk_flg>"
  Bus Selector6/34 -> CANInvalidDetection/34(VINP_CANMsgEPAS2RolCntOk_flg)  "<VINP_CANMsgEPAS2RolCntOk_flg>"
  Bus Selector6/35 -> CANInvalidDetection/35(VINP_CANMsgEPAS3RolCntOk_flg)  "<VINP_CANMsgEPAS3RolCntOk_flg>"
  Bus Selector6/36 -> CANInvalidDetection/36(VINP_CANMsgVCU1RolCntOk_flg)  "<VINP_CANMsgVCU1RolCntOk_flg>"
  Bus Selector6/37 -> CANInvalidDetection/37(VINP_CANMsgVCU2RolCntOk_flg)  "<VINP_CANMsgVCU2RolCntOk_flg>"
  Bus Selector6/38 -> CANInvalidDetection/38(VINP_CANMsgHCU3RolCntOk_flg)  "<VINP_CANMsgHCU3RolCntOk_flg>"
  Bus Selector6/39 -> CANInvalidDetection/39(VINP_CANMsgABS2ChkSumOk_flg)  "<VINP_CANMsgABS2ChkSumOk_flg>"
  Bus Selector6/40 -> CANInvalidDetection/40(VINP_CANMsgSAS1ChkSumOk_flg)  "<VINP_CANMsgSAS1ChkSumOk_flg>"
  Bus Selector6/41 -> CANInvalidDetection/41(VINP_CANMsgEPAS2ChkSumOk_flg)  "<VINP_CANMsgEPAS2ChkSumOk_flg>"
  Bus Selector6/42 -> CANInvalidDetection/42(VINP_CANMsgEPAS3ChkSumOk_flg)  "<VINP_CANMsgEPAS3ChkSumOk_flg>"
  Bus Selector6/43 -> CANInvalidDetection/43(VINP_CANMsgVCU1ChkSumOk_flg)  "<VINP_CANMsgVCU1ChkSumOk_flg>"
  Bus Selector6/44 -> CANInvalidDetection/44(VINP_CANMsgVCU2ChkSumOk_flg)  "<VINP_CANMsgVCU2ChkSumOk_flg>"
  [CANvalid_bus] -> Bus Selector4/1
  Bus Selector4/3 -> CANInvalidDetection_byType/3(VINP_CANMsgECM9RolCntOk_flg)  "<VINP_CANMsgECM9RolCntOk_flg>"
  Bus Selector4/4 -> CANInvalidDetection_byType/4(VINP_CANMsgSAS1RolCntOk_flg)  "<VINP_CANMsgSAS1RolCntOk_flg>"
  Bus Selector4/2 -> CANInvalidDetection_byType/2(VINP_CANMsgABS2RolCntOk_flg)  "<VINP_CANMsgABS2RolCntOk_flg>"
  Bus Selector4/5 -> CANInvalidDetection_byType/5(VINP_CANMsgEPAS2RolCntOk_flg)  "<VINP_CANMsgEPAS2RolCntOk_flg>"
  Bus Selector4/6 -> CANInvalidDetection_byType/6(VINP_CANMsgEPAS3RolCntOk_flg)  "<VINP_CANMsgEPAS3RolCntOk_flg>"
  Bus Selector4/1 -> CANInvalidDetection_byType/1(VINP_CANMsgABS1RolCntOk_flg)  "<VINP_CANMsgABS1RolCntOk_flg>"
  Bus Selector4/7 -> CANInvalidDetection_byType/7(VINP_CANMsgVCU1RolCntOk_flg)  "<VINP_CANMsgVCU1RolCntOk_flg>"
  Bus Selector4/8 -> CANInvalidDetection_byType/8(VINP_CANMsgVCU2RolCntOk_flg)  "<VINP_CANMsgVCU2RolCntOk_flg>"
  Bus Selector4/9 -> CANInvalidDetection_byType/9(VINP_CANMsgHCU3RolCntOk_flg)  "<VINP_CANMsgHCU3RolCntOk_flg>"
  Bus Selector4/10 -> CANInvalidDetection_byType/10(VINP_CANMsgABS2ChkSumOk_flg)  "<VINP_CANMsgABS2ChkSumOk_flg>"
  Bus Selector4/11 -> CANInvalidDetection_byType/11(VINP_CANMsgSAS1ChkSumOk_flg)  "<VINP_CANMsgSAS1ChkSumOk_flg>"
  Bus Selector4/12 -> CANInvalidDetection_byType/12(VINP_CANMsgEPAS2ChkSumOk_flg)  "<VINP_CANMsgEPAS2ChkSumOk_flg>"
  Bus Selector4/13 -> CANInvalidDetection_byType/13(VINP_CANMsgEPAS3ChkSumOk_flg)  "<VINP_CANMsgEPAS3ChkSumOk_flg>"
  Bus Selector4/14 -> CANInvalidDetection_byType/14(VINP_CANMsgVCU1ChkSumOk_flg)  "<VINP_CANMsgVCU1ChkSumOk_flg>"
  Bus Selector4/15 -> CANInvalidDetection_byType/15(VINP_CANMsgVCU2ChkSumOk_flg)  "<VINP_CANMsgVCU2ChkSumOk_flg>"
  [ECM_signal_bus] -> Bus Selector9/1
  Bus Selector9/1 -> ECM_CANSig_Detection/1(VINP_AccelPedalPosnSigOk_flg_InHAL)  "<VINP_AccelPedalPosnSigOk_flg_InHAL>"
  [ABS_signal_bus] -> Bus Selector11/1
  Bus Selector11/1 -> ABS_CANSig_Detection/1(VINP_BrkLampOnSigOk_flg_InHAL)  "<VINP_BrkLampOnSigOk_flg_InHAL>"
  Bus Selector11/2 -> ABS_CANSig_Detection/2(VINP_VehSpdSigOk_flg_InHAL)  "<VINP_VehSpdSigOk_flg_InHAL>"
  Bus Selector11/3 -> ABS_CANSig_Detection/3(VINP_YawRateSigOk_flg_InHAL)  "<VINP_YawRateSigOk_flg_InHAL>"
  [EPS_signal_bus] -> Bus Selector14/1
  Bus Selector14/1 -> EPS_CANSig_Detection/1(VINP_SteerAngleSigOk_flg_InHAL)  "<VINP_SteerAngleSigOk_flg_InHAL>"
  Bus Selector9/2 -> ECM_CANSig_Detection/2(VINP_EffAccelPedalPosnSigOk_flg_InHAL)  "<VINP_EffAccelPedalPosnSigOk_flg_InHAL>"
  Bus Selector9/3 -> ECM_CANSig_Detection/3(VINP_EngSpdSigOk_flg_InHAL)  "<VINP_EngSpdSigOk_flg_InHAL>"
  Bus Selector9/4 -> ECM_CANSig_Detection/4(VINP_EngTqFrictionSigOk_flg_InHAL)  "<VINP_EngTqFrictionSigOk_flg_InHAL>"
  Bus Selector9/7 -> ECM_CANSig_Detection/7(VINP_EngTqMaxSigOk_flg_InHAL)  "<VINP_EngTqMaxSigOk_flg_InHAL>"
  Bus Selector9/8 -> ECM_CANSig_Detection/8(VINP_EngTqMinSigOk_flg_InHAL)  "<VINP_EngTqMinSigOk_flg_InHAL>"
  Bus Selector11/4 -> ABS_CANSig_Detection/4(VINP_LatAccelSigOk_flg_InHAL)  "<VINP_LatAccelSigOk_flg_InHAL>"
  Bus Selector11/5 -> ABS_CANSig_Detection/5(VINP_LongAccelSigOk_flg_InHAL)  "<VINP_LongAccelSigOk_flg_InHAL>"
  Bus Selector11/6 -> ABS_CANSig_Detection/6(VINP_MCPressureSigOk_flg_InHAL)  "<VINP_MCPressureSigOk_flg_InHAL>"
  Bus Selector14/4 -> EPS_CANSig_Detection/4(VINP_SteerTorqueSigOk_flg_InHAL)  "<VINP_SteerTorqueSigOk_flg_InHAL>"
  Bus Selector9/5 -> ECM_CANSig_Detection/5(VINP_EngTqIndicatedSigOk_flg_InHAL)  "<VINP_EngTqIndicatedSigOk_flg_InHAL>"
  Bus Selector9/6 -> ECM_CANSig_Detection/6(VINP_EngTqIndicatedCORSigOk_flg_InHAL)  "<VINP_EngTqIndicatedCORSigOk_flg_InHAL>"
  Bus Selector11/7 -> ABS_CANSig_Detection/7(VINP_SurfaceGradeSigOk_flg_InHAL)  "<VINP_SurfaceGradeSigOk_flg_InHAL>"
  Bus Selector11/8 -> ABS_CANSig_Detection/8(VINP_BrakBoosterPresSigOk_flg_InHAL)  "<VINP_BrakBoosterPresSigOk_flg_InHAL>"
  Bus Selector11/9 -> ABS_CANSig_Detection/9(VINP_LFWheelSpeedSigOk_flg_InHAL)  "<VINP_LFWheelSpeedSigOk_flg_InHAL>"
  Bus Selector11/10 -> ABS_CANSig_Detection/10(VINP_LRWheelSpeedSigOk_flg_InHAL)  "<VINP_LRWheelSpeedSigOk_flg_InHAL>"
  Bus Selector11/11 -> ABS_CANSig_Detection/11(VINP_RFWheelSpeedSigOk_flg_InHAL)  "<VINP_RFWheelSpeedSigOk_flg_InHAL>"
  Bus Selector11/12 -> ABS_CANSig_Detection/12(VINP_RRWheelSpeedSigOk_flg_InHAL)  "<VINP_RRWheelSpeedSigOk_flg_InHAL>"
  Bus Selector14/3 -> EPS_CANSig_Detection/3(VINP_RelSteerAngleSigOk_flg_InHAL)  "<VINP_RelSteerAngleSigOk_flg_InHAL>"
  Bus Selector14/5 -> EPS_CANSig_Detection/5(VINP_AckCurStrAngReqSigOk_flg_InHAL)  "<VINP_AckCurStrAngReqSigOk_flg_InHAL>"
  Bus Selector14/6 -> EPS_CANSig_Detection/6(VINP_AckCurStrTqReqSigOk_flg_InHAL)  "<VINP_AckCurStrTqReqSigOk_flg_InHAL>"
  Bus Selector14/7 -> EPS_CANSig_Detection/7(VINP_EPSBattVoltSigOk_flg_InHAL)  "<VINP_EPSBattVoltSigOk_flg_InHAL>"
  [BCM_signal_bus] -> Bus Selector2/1
  Bus Selector2/1 -> BCM_CANSig_Detection/1(VINP_VehCrashedSigOk_flg_InHAL)  "<VINP_VehCrashedSigOk_flg_InHAL>"
  [TCM_signal_bus] -> Bus Selector3/1
  Bus Selector3/1 -> TCM_CANSig_Detection/1(VINP_ShifterPosnSigOk_flg_InHAL)  "<VINP_ShifterPosnSigOk_flg_InHAL>"
  Bus Selector3/2 -> TCM_CANSig_Detection/2(VINP_NowGearPosnSigOk_flg_InHAL)  "<VINP_NowGearPosnSigOk_flg_InHAL>"
  Bus Selector3/3 -> TCM_CANSig_Detection/3(VINP_TMTurbineSpdSigOk_flg_InHAL)  "<VINP_TMTurbineSpdSigOk_flg_InHAL>"
  [AC_signal_bus] -> Bus Selector10/1
  Bus Selector10/1 -> AC_CANSig_Detection/1(VINP_OutsideTempSigOk_flg_InHAL)  "<VINP_OutsideTempSigOk_flg_InHAL>"
  [METER_signal_bus] -> Bus Selector15/1
  Bus Selector15/1 -> METER_CANSig_Detection/1(VINP_OdoMeterSigOk_flg_InHAL)  "<VINP_OdoMeterSigOk_flg_InHAL>"
  Bus Selector15/2 -> METER_CANSig_Detection/2(VINP_MeterVehSpdSigOk_flg_InHAL)  "<VINP_MeterVehSpdSigOk_flg_InHAL>"
  [EPB_signal_bus] -> Bus Selector16/1
  Bus Selector16/1 -> EPB_CANSig_Detection/1(VINP_ParkBrakeBindStaSigOk_flg_InHAL)  "<VINP_ParkBrakeBindStaSigOk_flg_InHAL>"
  [VCU_signal_bus] -> Bus Selector17/1
  Bus Selector17/1 -> VCU_CANSig_Detection/1(VINP_AckCurBrakDecReqSigOk_flg_InHAL)  "<VINP_AckCurBrakDecReqSigOk_flg_InHAL>"
  Bus Selector17/2 -> VCU_CANSig_Detection/2(VINP_AckCurPTTqReqSigOk_flg_InHAL)  "<VINP_AckCurPTTqReqSigOk_flg_InHAL>"
  Bus Selector17/3 -> VCU_CANSig_Detection/3(VINP_AckCurVehDecSigOk_flg_InHAL)  "<VINP_AckCurVehDecSigOk_flg_InHAL>"
  Bus Selector17/5 -> VCU_CANSig_Detection/5(VINP_ESCBattVoltSigOk_flg_InHAL)  "<VINP_ESCBattVoltSigOk_flg_InHAL>"
  Bus Selector17/6 -> VCU_CANSig_Detection/6(VINP_HWAccelPedalPosnSigOk_flg_InHAL)  "<VINP_HWAccelPedalPosnSigOk_flg_InHAL>"
  [VINP_EPSCANAlive_flg_InProc] -> inputs_bus2/17
  [VINP_TCMCANAlive_flg_InProc] -> inputs_bus2/16
  [VINP_ECMCANAlive_flg_InProc] -> inputs_bus2/15
  [VINP_BCMCANAlive_flg_InProc] -> inputs_bus2/14
  [VINP_ABSCANAlive_flg_InProc] -> inputs_bus2/13
  [VINP_EPSCANValid_flg_InProc] -> inputs_bus2/5
  [VINP_TCMCANValid_flg_InProc] -> inputs_bus2/4
  [VINP_ECMCANValid_flg_InProc] -> inputs_bus2/3
  [VINP_BCMCANValid_flg_InProc] -> inputs_bus2/2
  [VINP_ABSCANValid_flg_InProc] -> inputs_bus2/1
  [VINP_METERCANValid_flg_InProc] -> inputs_bus2/6
  [VINP_METERCANAlive_flg_InProc] -> inputs_bus2/18
  [VINP_ACCANValid_flg_InProc] -> inputs_bus2/7
  [VINP_EPBCANValid_flg_InProc] -> inputs_bus2/8
  [VINP_VCUCANValid_flg_InProc] -> inputs_bus2/9
  [VINP_ACCANAlive_flg_InProc] -> inputs_bus2/19
  [VINP_EPBCANAlive_flg_InProc] -> inputs_bus2/20
  [VINP_VCUCANAlive_flg_InProc] -> inputs_bus2/21
  inputs_bus2/1 -> INP_diag_bus/1
  [VINP_HMICANValid_flg_InProc] -> inputs_bus2/10
  [VINP_EPMCANValid_flg_InProc] -> inputs_bus2/12
  [VINP_HMICANAlive_flg_InProc] -> inputs_bus2/22
  [VINP_EPMCANAlive_flg_InProc] -> inputs_bus2/24
  Bus Selector26/14 -> CANCommunicationDetection/14(VINP_CANMsgEPAS1CommuOk_flg)  "<VINP_CANMsgEPAS1CommuOk_flg>"
  Bus Selector1/14 -> Proc_CANAlive/14(VINP_CANMsgEPAS1CommuOk_flg)  "<VINP_CANMsgEPAS1CommuOk_flg>"
  Bus Selector5/14 -> CANCommunicationDetection_byID/14(VINP_CANMsgEPAS1CommuOk_flg)  "<VINP_CANMsgEPAS1CommuOk_flg>"
  Bus Selector6/14 -> CANInvalidDetection/14(VINP_CANMsgEPAS1CommuOk_flg)  "<VINP_CANMsgEPAS1CommuOk_flg>"
  Bus Selector14/2 -> EPS_CANSig_Detection/2(VINP_SteerSpeedSigOk_flg_InHAL)  "<VINP_SteerSpeedSigOk_flg_InHAL>"
  Bus Selector17/7 -> VCU_CANSig_Detection/7(VINP_CurDriverTqReqSigOk_flg_InHAL)  "<VINP_CurDriverTqReqSigOk_flg_InHAL>"
  Bus Selector26/27 -> CANCommunicationDetection/27(VINP_CANMsgCSW1CommuOk_flg)  "<VINP_CANMsgCSW1CommuOk_flg>"
  CANCommunicationDetection/11(VINP_CSWCANValid_flg_InProc) -> [VINP_CSWCANValid_flg_InProc]
  [VINP_CSWCANValid_flg_InProc] -> inputs_bus2/11
  Bus Selector1/27 -> Proc_CANAlive/27(VINP_CANMsgCSW1CommuOk_flg)  "<VINP_CANMsgCSW1CommuOk_flg>"
  Proc_CANAlive/11(VINP_CSWCANAlive_flg_InProc) -> [VINP_CSWCANAlive_flg_InProc]
  [VINP_CSWCANAlive_flg_InProc] -> inputs_bus2/23
  Bus Selector5/27 -> CANCommunicationDetection_byID/27(VINP_CANMsgCSW1CommuOk_flg)  "<VINP_CANMsgCSW1CommuOk_flg>"
  Bus Selector6/27 -> CANInvalidDetection/27(VINP_CANMsgCSW1CommuOk_flg)  "<VINP_CANMsgCSW1CommuOk_flg>"
  [CSW_signal_bus] -> Terminator5/1
  CSW_signal_bus/1 -> [CSW_signal_bus]
  Bus Selector17/4 -> VCU_CANSig_Detection/4(VINP_DriverBrakPedalOnSigOk_flg_InHAL)  "<VINP_DriverBrakPedalOnSigOk_flg_InHAL>"
  Bus Selector4/16 -> CANInvalidDetection_byType/16(VINP_CamCANReceiveOk_flg)  "<VINP_CamCANReceiveOk_flg>"
